simulink model "isi_correlator_tvg"  (Simulink 7.3)
settings: Solver=ode45  StartTime=0.0  StopTime=10.0  RelTol=1e-3
undefined symbols (not defined in the file or in its folder): pfb_coeff_gen_calc, ROACH
libraries: xbsIndex_r4 x5206, casper_library x454, isi_correlator_lib x28, dspsigops x16, xps_library x14
(part 2 of 51)

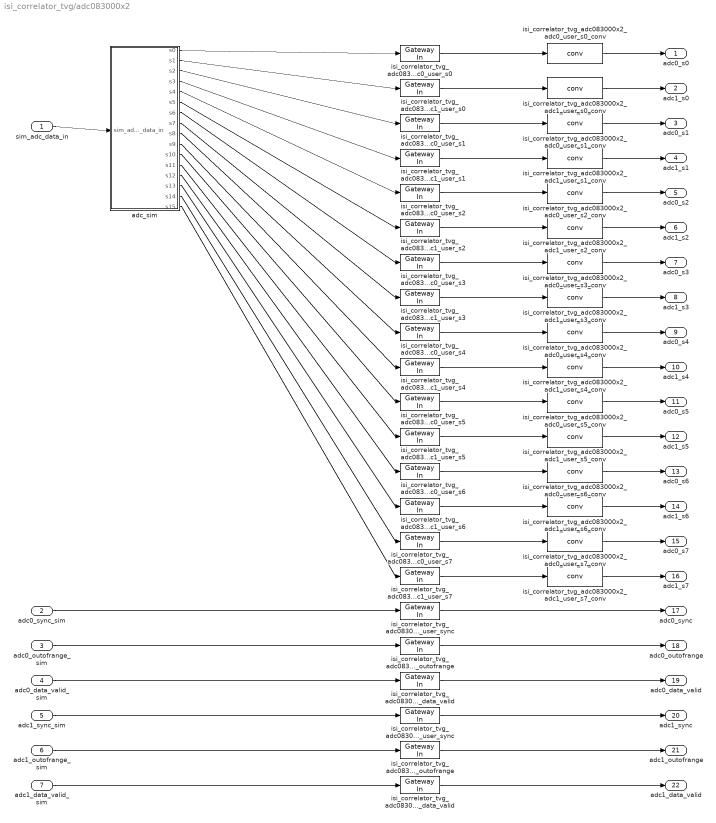
== system isi_correlator_tvg/adc083000x2 ==
  sim_adc_data_in : Inport  OutDataType=sfix(16)  OutScaling=2^0
  adc0_sync_sim : Inport  Port=2  OutDataType=sfix(16)  OutScaling=2^0
  adc0_outofrange_sim : Inport  Port=3  OutDataType=sfix(16)  OutScaling=2^0
  adc0_data_valid_sim : Inport  Port=4  OutDataType=sfix(16)  OutScaling=2^0
  adc1_sync_sim : Inport  Port=5  OutDataType=sfix(16)  OutScaling=2^0
  adc1_outofrange_sim : Inport  Port=6  OutDataType=sfix(16)  OutScaling=2^0
  adc1_data_valid_sim : Inport  Port=7  OutDataType=sfix(16)  OutScaling=2^0
  adc_sim : Subsystem  AncestorBlock=casper_library/Misc/adc_sim  mask(adc_sim){nStreams=16}
      mask prompts: nStreams "Number of Output Streams"
      mask help:
      | This block simulates the behavior of a demuxed ADC, which takes
      | as input a waveform and returns digital samples in parallel at
      | a slower rate.
      |
      | This block is used to simulate the behavior
      mask init:
      | adc_sim_init(gcb, ...
      |     'nStreams', nStreams );
  isi_correlator_tvg_adc083000x2_adc0_user_data_valid : xbsIndex_r4/Gateway In  arith_type=Unsigned  n_bits=1  bin_pt=0  quantization="Round  (unbiased: +/- Inf)"  overflow=Saturate  period=1  dbl_ovrd=off  timing_constraint=None  locs_specified=off  LOCs={}  block_type=gatewayin
      settings: infoedit="Gateway in block.  Converts inputs of type Simulink integer, double and fixed point to  Xilinx fixed point type.<br><br>Hardware notes:  In hardware these blocks become top level input ports."  block_version=11.3
  isi_correlator_tvg_adc083000x2_adc0_user_outofrange : xbsIndex_r4/Gateway In  arith_type=Unsigned  n_bits=4  bin_pt=0  quantization="Round  (unbiased: +/- Inf)"  overflow=Saturate  period=1  dbl_ovrd=off  timing_constraint=None  locs_specified=off  LOCs={}  block_type=gatewayin
      settings: infoedit="Gateway in block.  Converts inputs of type Simulink integer, double and fixed point to  Xilinx fixed point type.<br><br>Hardware notes:  In hardware these blocks become top level input ports."  block_version=11.3
  isi_correlator_tvg_adc083000x2_adc0_user_s{0..7} (8 alike) : xbsIndex_r4/Gateway In  arith_type=Unsigned  n_bits=8  bin_pt=0  quantization="Round  (unbiased: +/- Inf)"  overflow=Saturate  period=1  dbl_ovrd=off  timing_constraint=None  locs_specified=off  LOCs={}  block_type=gatewayin
      settings: infoedit="Gateway in block.  Converts inputs of type Simulink integer, double and fixed point to  Xilinx fixed point type.<br><br>Hardware notes:  In hardware these blocks become top level input ports."  block_version=11.3
  isi_correlator_tvg_adc083000x2_adc0_user_s0_conv : casper_library/Misc/conv  SystemSampleTime=-1
  isi_correlator_tvg_adc083000x2_adc0_user_s1_conv : casper_library/Misc/conv  SystemSampleTime=-1
  isi_correlator_tvg_adc083000x2_adc0_user_s2_conv : casper_library/Misc/conv  SystemSampleTime=-1
  isi_correlator_tvg_adc083000x2_adc0_user_s3_conv : casper_library/Misc/conv  SystemSampleTime=-1
  isi_correlator_tvg_adc083000x2_adc0_user_s4_conv : casper_library/Misc/conv  SystemSampleTime=-1
  isi_correlator_tvg_adc083000x2_adc0_user_s5_conv : casper_library/Misc/conv  SystemSampleTime=-1
  isi_correlator_tvg_adc083000x2_adc0_user_s6_conv : casper_library/Misc/conv  SystemSampleTime=-1
  isi_correlator_tvg_adc083000x2_adc0_user_s7_conv : casper_library/Misc/conv  SystemSampleTime=-1
  isi_correlator_tvg_adc083000x2_adc0_user_sync : xbsIndex_r4/Gateway In  arith_type=Unsigned  n_bits=4  bin_pt=0  quantization="Round  (unbiased: +/- Inf)"  overflow=Saturate  period=1  dbl_ovrd=off  timing_constraint=None  locs_specified=off  LOCs={}  block_type=gatewayin
      settings: infoedit="Gateway in block.  Converts inputs of type Simulink integer, double and fixed point to  Xilinx fixed point type.<br><br>Hardware notes:  In hardware these blocks become top level input ports."  block_version=11.3
  isi_correlator_tvg_adc083000x2_adc1_user_data_valid : xbsIndex_r4/Gateway In  arith_type=Unsigned  n_bits=1  bin_pt=0  quantization="Round  (unbiased: +/- Inf)"  overflow=Saturate  period=1  dbl_ovrd=off  timing_constraint=None  locs_specified=off  LOCs={}  block_type=gatewayin
      settings: infoedit="Gateway in block.  Converts inputs of type Simulink integer, double and fixed point to  Xilinx fixed point type.<br><br>Hardware notes:  In hardware these blocks become top level input ports."  block_version=11.3
  isi_correlator_tvg_adc083000x2_adc1_user_outofrange : xbsIndex_r4/Gateway In  arith_type=Unsigned  n_bits=4  bin_pt=0  quantization="Round  (unbiased: +/- Inf)"  overflow=Saturate  period=1  dbl_ovrd=off  timing_constraint=None  locs_specified=off  LOCs={}  block_type=gatewayin
      settings: infoedit="Gateway in block.  Converts inputs of type Simulink integer, double and fixed point to  Xilinx fixed point type.<br><br>Hardware notes:  In hardware these blocks become top level input ports."  block_version=11.3
  isi_correlator_tvg_adc083000x2_adc1_user_s{0..7} (8 alike) : xbsIndex_r4/Gateway In  arith_type=Unsigned  n_bits=8  bin_pt=0  quantization="Round  (unbiased: +/- Inf)"  overflow=Saturate  period=1  dbl_ovrd=off  timing_constraint=None  locs_specified=off  LOCs={}  block_type=gatewayin
      settings: infoedit="Gateway in block.  Converts inputs of type Simulink integer, double and fixed point to  Xilinx fixed point type.<br><br>Hardware notes:  In hardware these blocks become top level input ports."  block_version=11.3
  isi_correlator_tvg_adc083000x2_adc1_user_s0_conv : casper_library/Misc/conv  SystemSampleTime=-1
  isi_correlator_tvg_adc083000x2_adc1_user_s1_conv : casper_library/Misc/conv  SystemSampleTime=-1
  isi_correlator_tvg_adc083000x2_adc1_user_s2_conv : casper_library/Misc/conv  SystemSampleTime=-1
  isi_correlator_tvg_adc083000x2_adc1_user_s3_conv : casper_library/Misc/conv  SystemSampleTime=-1
  isi_correlator_tvg_adc083000x2_adc1_user_s4_conv : casper_library/Misc/conv  SystemSampleTime=-1
  isi_correlator_tvg_adc083000x2_adc1_user_s5_conv : casper_library/Misc/conv  SystemSampleTime=-1
  isi_correlator_tvg_adc083000x2_adc1_user_s6_conv : casper_library/Misc/conv  SystemSampleTime=-1
  isi_correlator_tvg_adc083000x2_adc1_user_s7_conv : casper_library/Misc/conv  SystemSampleTime=-1
  isi_correlator_tvg_adc083000x2_adc1_user_sync : xbsIndex_r4/Gateway In  arith_type=Unsigned  n_bits=4  bin_pt=0  quantization="Round  (unbiased: +/- Inf)"  overflow=Saturate  period=1  dbl_ovrd=off  timing_constraint=None  locs_specified=off  LOCs={}  block_type=gatewayin
      settings: infoedit="Gateway in block.  Converts inputs of type Simulink integer, double and fixed point to  Xilinx fixed point type.<br><br>Hardware notes:  In hardware these blocks become top level input ports."  block_version=11.3
  adc0_s0 : Outport  OutDataType=fixdt(1, 16)  OutScaling=2^0
  adc1_s0 : Outport  Port=2  OutDataType=fixdt(1, 16)  OutScaling=2^0
  adc0_s1 : Outport  Port=3  OutDataType=fixdt(1, 16)  OutScaling=2^0
  adc1_s1 : Outport  Port=4  OutDataType=fixdt(1, 16)  OutScaling=2^0
  adc0_s2 : Outport  Port=5  OutDataType=fixdt(1, 16)  OutScaling=2^0
  adc1_s2 : Outport  Port=6  OutDataType=fixdt(1, 16)  OutScaling=2^0
  adc0_s3 : Outport  Port=7  OutDataType=fixdt(1, 16)  OutScaling=2^0
  adc1_s3 : Outport  Port=8  OutDataType=fixdt(1, 16)  OutScaling=2^0
  adc0_s4 : Outport  Port=9  OutDataType=fixdt(1, 16)  OutScaling=2^0
  adc1_s4 : Outport  Port=10  OutDataType=fixdt(1, 16)  OutScaling=2^0
  adc0_s5 : Outport  Port=11  OutDataType=fixdt(1, 16)  OutScaling=2^0
  adc1_s5 : Outport  Port=12  OutDataType=fixdt(1, 16)  OutScaling=2^0
  adc0_s6 : Outport  Port=13  OutDataType=fixdt(1, 16)  OutScaling=2^0
  adc1_s6 : Outport  Port=14  OutDataType=fixdt(1, 16)  OutScaling=2^0
  adc0_s7 : Outport  Port=15  OutDataType=fixdt(1, 16)  OutScaling=2^0
  adc1_s7 : Outport  Port=16  OutDataType=fixdt(1, 16)  OutScaling=2^0
  adc0_sync : Outport  Port=17  OutDataType=sfix(16)  OutScaling=2^0
  adc0_outofrange : Outport  Port=18  OutDataType=sfix(16)  OutScaling=2^0
  adc0_data_valid : Outport  Port=19  OutDataType=sfix(16)  OutScaling=2^0
  adc1_sync : Outport  Port=20  OutDataType=sfix(16)  OutScaling=2^0
  adc1_outofrange : Outport  Port=21  OutDataType=sfix(16)  OutScaling=2^0
  adc1_data_valid : Outport  Port=22  OutDataType=sfix(16)  OutScaling=2^0
  wires:
  sim_adc_data_in/1 -> adc_sim/1(sim_adc_data_in)
  adc_sim/1(s0) -> isi_correlator_tvg_adc083000x2_adc0_user_s0/1
  isi_correlator_tvg_adc083000x2_adc0_user_s0/1 -> isi_correlator_tvg_adc083000x2_adc0_user_s0_conv/1
  isi_correlator_tvg_adc083000x2_adc0_user_s0_conv/1 -> adc0_s0/1
  adc_sim/2(s1) -> isi_correlator_tvg_adc083000x2_adc1_user_s0/1
  isi_correlator_tvg_adc083000x2_adc1_user_s0/1 -> isi_correlator_tvg_adc083000x2_adc1_user_s0_conv/1
  isi_correlator_tvg_adc083000x2_adc1_user_s0_conv/1 -> adc1_s0/1
  adc_sim/3(s2) -> isi_correlator_tvg_adc083000x2_adc0_user_s1/1
  isi_correlator_tvg_adc083000x2_adc0_user_s1/1 -> isi_correlator_tvg_adc083000x2_adc0_user_s1_conv/1
  isi_correlator_tvg_adc083000x2_adc0_user_s1_conv/1 -> adc0_s1/1
  adc_sim/4(s3) -> isi_correlator_tvg_adc083000x2_adc1_user_s1/1
  isi_correlator_tvg_adc083000x2_adc1_user_s1/1 -> isi_correlator_tvg_adc083000x2_adc1_user_s1_conv/1
  isi_correlator_tvg_adc083000x2_adc1_user_s1_conv/1 -> adc1_s1/1
  adc_sim/5(s4) -> isi_correlator_tvg_adc083000x2_adc0_user_s2/1
  isi_correlator_tvg_adc083000x2_adc0_user_s2/1 -> isi_correlator_tvg_adc083000x2_adc0_user_s2_conv/1
  isi_correlator_tvg_adc083000x2_adc0_user_s2_conv/1 -> adc0_s2/1
  adc_sim/6(s5) -> isi_correlator_tvg_adc083000x2_adc1_user_s2/1
  isi_correlator_tvg_adc083000x2_adc1_user_s2/1 -> isi_correlator_tvg_adc083000x2_adc1_user_s2_conv/1
  isi_correlator_tvg_adc083000x2_adc1_user_s2_conv/1 -> adc1_s2/1
  adc_sim/7(s6) -> isi_correlator_tvg_adc083000x2_adc0_user_s3/1
  isi_correlator_tvg_adc083000x2_adc0_user_s3/1 -> isi_correlator_tvg_adc083000x2_adc0_user_s3_conv/1
  isi_correlator_tvg_adc083000x2_adc0_user_s3_conv/1 -> adc0_s3/1
  adc_sim/8(s7) -> isi_correlator_tvg_adc083000x2_adc1_user_s3/1
  isi_correlator_tvg_adc083000x2_adc1_user_s3/1 -> isi_correlator_tvg_adc083000x2_adc1_user_s3_conv/1
  isi_correlator_tvg_adc083000x2_adc1_user_s3_conv/1 -> adc1_s3/1
  adc_sim/9(s8) -> isi_correlator_tvg_adc083000x2_adc0_user_s4/1
  isi_correlator_tvg_adc083000x2_adc0_user_s4/1 -> isi_correlator_tvg_adc083000x2_adc0_user_s4_conv/1
  isi_correlator_tvg_adc083000x2_adc0_user_s4_conv/1 -> adc0_s4/1
  adc_sim/10(s9) -> isi_correlator_tvg_adc083000x2_adc1_user_s4/1
  isi_correlator_tvg_adc083000x2_adc1_user_s4/1 -> isi_correlator_tvg_adc083000x2_adc1_user_s4_conv/1
  isi_correlator_tvg_adc083000x2_adc1_user_s4_conv/1 -> adc1_s4/1
  adc_sim/11(s10) -> isi_correlator_tvg_adc083000x2_adc0_user_s5/1
  isi_correlator_tvg_adc083000x2_adc0_user_s5/1 -> isi_correlator_tvg_adc083000x2_adc0_user_s5_conv/1
  isi_correlator_tvg_adc083000x2_adc0_user_s5_conv/1 -> adc0_s5/1
  adc_sim/12(s11) -> isi_correlator_tvg_adc083000x2_adc1_user_s5/1
  isi_correlator_tvg_adc083000x2_adc1_user_s5/1 -> isi_correlator_tvg_adc083000x2_adc1_user_s5_conv/1
  isi_correlator_tvg_adc083000x2_adc1_user_s5_conv/1 -> adc1_s5/1
  adc_sim/13(s12) -> isi_correlator_tvg_adc083000x2_adc0_user_s6/1
  isi_correlator_tvg_adc083000x2_adc0_user_s6/1 -> isi_correlator_tvg_adc083000x2_adc0_user_s6_conv/1
  isi_correlator_tvg_adc083000x2_adc0_user_s6_conv/1 -> adc0_s6/1
  adc_sim/14(s13) -> isi_correlator_tvg_adc083000x2_adc1_user_s6/1
  isi_correlator_tvg_adc083000x2_adc1_user_s6/1 -> isi_correlator_tvg_adc083000x2_adc1_user_s6_conv/1
  isi_correlator_tvg_adc083000x2_adc1_user_s6_conv/1 -> adc1_s6/1
  adc_sim/15(s14) -> isi_correlator_tvg_adc083000x2_adc0_user_s7/1
  isi_correlator_tvg_adc083000x2_adc0_user_s7/1 -> isi_correlator_tvg_adc083000x2_adc0_user_s7_conv/1
  isi_correlator_tvg_adc083000x2_adc0_user_s7_conv/1 -> adc0_s7/1
  adc_sim/16(s15) -> isi_correlator_tvg_adc083000x2_adc1_user_s7/1
  isi_correlator_tvg_adc083000x2_adc1_user_s7/1 -> isi_correlator_tvg_adc083000x2_adc1_user_s7_conv/1
  isi_correlator_tvg_adc083000x2_adc1_user_s7_conv/1 -> adc1_s7/1
  adc0_sync_sim/1 -> isi_correlator_tvg_adc083000x2_adc0_user_sync/1
  isi_correlator_tvg_adc083000x2_adc0_user_sync/1 -> adc0_sync/1
  adc0_outofrange_sim/1 -> isi_correlator_tvg_adc083000x2_adc0_user_outofrange/1
  isi_correlator_tvg_adc083000x2_adc0_user_outofrange/1 -> adc0_outofrange/1
  adc0_data_valid_sim/1 -> isi_correlator_tvg_adc083000x2_adc0_user_data_valid/1
  isi_correlator_tvg_adc083000x2_adc0_user_data_valid/1 -> adc0_data_valid/1
  adc1_sync_sim/1 -> isi_correlator_tvg_adc083000x2_adc1_user_sync/1
  isi_correlator_tvg_adc083000x2_adc1_user_sync/1 -> adc1_sync/1
  adc1_outofrange_sim/1 -> isi_correlator_tvg_adc083000x2_adc1_user_outofrange/1
  isi_correlator_tvg_adc083000x2_adc1_user_outofrange/1 -> adc1_outofrange/1
  adc1_data_valid_sim/1 -> isi_correlator_tvg_adc083000x2_adc1_user_data_valid/1
  isi_correlator_tvg_adc083000x2_adc1_user_data_valid/1 -> adc1_data_valid/1

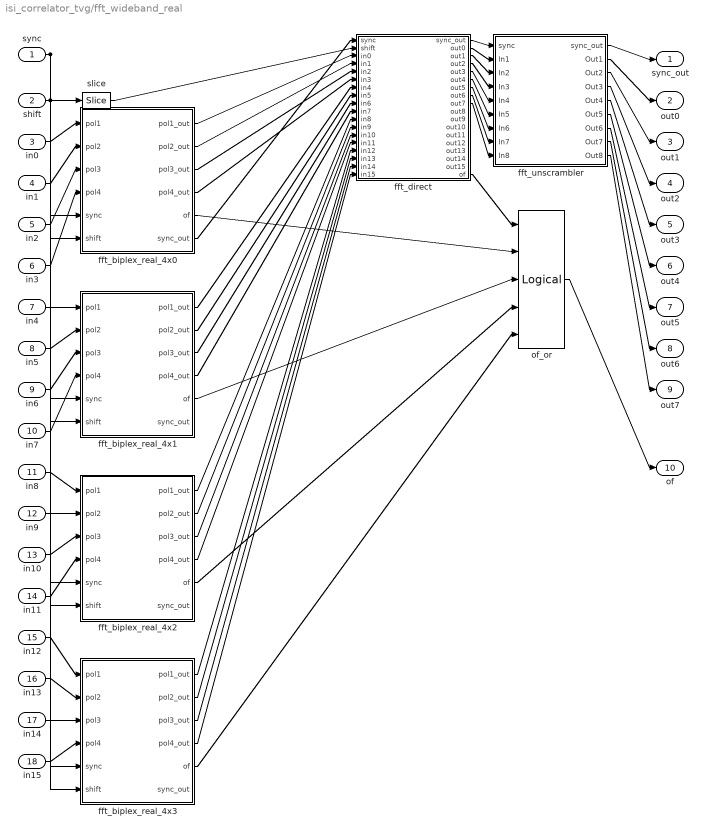
== system isi_correlator_tvg/fft_wideband_real ==
  sync : Inport  OutDataType=sfix(16)  OutScaling=2^0
  shift : Inport  Port=2  OutDataType=sfix(16)  OutScaling=2^0
  in0 : Inport  Port=3  OutDataType=sfix(16)  OutScaling=2^0
  in1 : Inport  Port=4  OutDataType=sfix(16)  OutScaling=2^0
  in2 : Inport  Port=5  OutDataType=sfix(16)  OutScaling=2^0
  in3 : Inport  Port=6  OutDataType=sfix(16)  OutScaling=2^0
  in4 : Inport  Port=7
  in5 : Inport  Port=8
  in6 : Inport  Port=9
  in7 : Inport  Port=10
  in8 : Inport  Port=11
  in9 : Inport  Port=12
  in10 : Inport  Port=13
  in11 : Inport  Port=14
  in12 : Inport  Port=15
  in13 : Inport  Port=16
  in14 : Inport  Port=17
  in15 : Inport  Port=18
  fft_biplex_real_4x0 : Subsystem  AncestorBlock=casper_library/FFTs/fft_biplex_real_4x  mask(fft_biplex_real_4x){FFTSize=3, input_bit_width=18, coeff_bit_width=18, quantization=Truncate, overflow=Wrap, add_latency=1, mult_latency=3, bram_latency=3, arch=Virtex5, opt_target=Multipliers, coeffs_bit_limit=8, delays_bit_limit=9, specify_mult=on, mult_spec=[1 1 1], dsp48_adders=on}
      mask prompts: FFTSize "Size of FFT: (2^? pnts)"; input_bit_width "Input/Output Bit Width"; coeff_bit_width "Coefficient Bit Width"; quantization "Quantization Behavior"; overflow "Overflow Behavior"; add_latency "Add Latency"; mult_latency "Mult Latency"; bram_latency "BRAM Latency"; arch "Architecture"; opt_target "Optimisation Target"; coeffs_bit_limit "Number bits above which to store stage's coefficients in BRAM (2^? bits)"; delays_bit_limit "Number bits above which to implement stage's delays in BRAM (2^? bits)"; specify_mult "Specify multiplier use (behavioral HDL if not specified)"; mult_spec "Multiplier specification (0=core, 1=embedded, 2=behavioural) (left=1st stage)"; dsp48_adders "Use DSP48s for adders"
      mask init:
      | fft_biplex_real_4x_init(gcb, ...
      |     'FFTSize', FFTSize, ...
      |     'input_bit_width', input_bit_width, ...
      |     'coeff_bit_width', coeff_bit_width, ...
      |     'quantization', quantization, ...
      |     'overflow', overflow, ...
      |     'add_latency', add_latency, ...
      |     'mult_latency', mult_latency, ...
      |     'bram_latency', bram_latency, ...
      |     'arch', arch, ...
      |     'opt_target', opt_target, ...
      |     'coeffs_bit_limit', coeffs_bit_limit, ...
      |     'delays_bit_limit', delays_bit_limit, ...    
      |     'specify_mult', specify_mult, ...
      |     'mult_spec', mult_spec, ...
      |     'dsp48_adders', dsp48_adders);
      settings: AttributesFormatString=FFTSize=3
      mask Help: eval('xlWeb([getenv(''MLIB_ROOT''), ''\doc\htmlblocks\sp_fft_biplex_real_4x\sp_fft_biplex_real_4x.html''])')
      mask StyleString: edit,edit,edit,popup(Truncate|Round  (unbiased: +/- Inf)|Round  (unbiased: Even Values)),popup(Wrap|Saturate|Error),edit,edit,edit,popup(Virtex2Pro|Virtex5),popup(Logic|Multipliers),edit,edit,checkbox,edit,checkbox
      mask CallbackString:
      | % fft_size = eval(get_param(gcb, 'FFTSize'));
      | % old_vec = eval(get_param(gcb, 'mult_spec'));
      | 
      | % vec = 2.*ones(1,fft_size);
      | % old_size = length(old_vec);
      | 
      | % if(old_size <= fft_size),
      | %     size = old_size;
      | % else,
      | %     size = fft_size;
      | % end
      | 
      | % for n = 1:size, vec(n) = old_vec(n); end
      | % set_param(gcb, 'mult_spec', mat2str(vec));||||||||||||specify_mult = get_param(gcb,'specify_mult');
      | visibs = get_param(gcb, 'MaskVisibilities');
      | enables = get_param(gcb, 'MaskEnables');
      | enables{14} = 'on';
      | if( strcmp(specify_mult,'off')), visibs{14} = 'off';
      | else, visibs{14} = 'on';
      | end
      | set_param(gcb, 'MaskVisibilities', visibs);
      | set_param(gcb, 'MaskEnables', enables);
      | |% vec = eval( get_param(gcb, 'mult_spec'));
      | % vec_size = length(vec);
      | % fft_size = eval( get_param(gcb, 'FFTSize'));
      | % new_vec = 2.*ones(1, fft_size);
      | 
      | % if(vec_size <= fft_size), size = vec_size;
      | % else size = fft_size;
      | % end
      | 
      | % for n=1:size
      | %    new_vec(n) = vec(n);
      | % end
      | % set_param(gcb, 'mult_spec', mat2str(new_vec));|
  fft_biplex_real_4x1 : Subsystem  AncestorBlock=casper_library/FFTs/fft_biplex_real_4x  mask(fft_biplex_real_4x){FFTSize=3, input_bit_width=18, coeff_bit_width=18, quantization=Truncate, overflow=Wrap, add_latency=1, mult_latency=3, bram_latency=3, arch=Virtex5, opt_target=Multipliers, coeffs_bit_limit=8, delays_bit_limit=9, specify_mult=on, mult_spec=[1 1 1], dsp48_adders=on}
      mask prompts: FFTSize "Size of FFT: (2^? pnts)"; input_bit_width "Input/Output Bit Width"; coeff_bit_width "Coefficient Bit Width"; quantization "Quantization Behavior"; overflow "Overflow Behavior"; add_latency "Add Latency"; mult_latency "Mult Latency"; bram_latency "BRAM Latency"; arch "Architecture"; opt_target "Optimisation Target"; coeffs_bit_limit "Number bits above which to store stage's coefficients in BRAM (2^? bits)"; delays_bit_limit "Number bits above which to implement stage's delays in BRAM (2^? bits)"; specify_mult "Specify multiplier use (behavioral HDL if not specified)"; mult_spec "Multiplier specification (0=core, 1=embedded, 2=behavioural) (left=1st stage)"; dsp48_adders "Use DSP48s for adders"
      mask init: same as isi_correlator_tvg/fft_wideband_real/fft_biplex_real_4x0
      settings: AttributesFormatString=FFTSize=3
      mask Help: eval('xlWeb([getenv(''MLIB_ROOT''), ''\doc\htmlblocks\sp_fft_biplex_real_4x\sp_fft_biplex_real_4x.html''])')
      mask StyleString: edit,edit,edit,popup(Truncate|Round  (unbiased: +/- Inf)|Round  (unbiased: Even Values)),popup(Wrap|Saturate|Error),edit,edit,edit,popup(Virtex2Pro|Virtex5),popup(Logic|Multipliers),edit,edit,checkbox,edit,checkbox
      mask CallbackString:
      | % fft_size = eval(get_param(gcb, 'FFTSize'));
      | % old_vec = eval(get_param(gcb, 'mult_spec'));
      | 
      | % vec = 2.*ones(1,fft_size);
      | % old_size = length(old_vec);
      | 
      | % if(old_size <= fft_size),
      | %     size = old_size;
      | % else,
      | %     size = fft_size;
      | % end
      | 
      | % for n = 1:size, vec(n) = old_vec(n); end
      | % set_param(gcb, 'mult_spec', mat2str(vec));||||||||||||specify_mult = get_param(gcb,'specify_mult');
      | visibs = get_param(gcb, 'MaskVisibilities');
      | enables = get_param(gcb, 'MaskEnables');
      | enables{14} = 'on';
      | if( strcmp(specify_mult,'off')), visibs{14} = 'off';
      | else, visibs{14} = 'on';
      | end
      | set_param(gcb, 'MaskVisibilities', visibs);
      | set_param(gcb, 'MaskEnables', enables);
      | |% vec = eval( get_param(gcb, 'mult_spec'));
      | % vec_size = length(vec);
      | % fft_size = eval( get_param(gcb, 'FFTSize'));
      | % new_vec = 2.*ones(1, fft_size);
      | 
      | % if(vec_size <= fft_size), size = vec_size;
      | % else size = fft_size;
      | % end
      | 
      | % for n=1:size
      | %    new_vec(n) = vec(n);
      | % end
      | % set_param(gcb, 'mult_spec', mat2str(new_vec));|
  fft_biplex_real_4x2 : Subsystem  AncestorBlock=casper_library/FFTs/fft_biplex_real_4x  mask(fft_biplex_real_4x){FFTSize=3, input_bit_width=18, coeff_bit_width=18, quantization=Truncate, overflow=Wrap, add_latency=1, mult_latency=3, bram_latency=3, arch=Virtex5, opt_target=Multipliers, coeffs_bit_limit=8, delays_bit_limit=9, specify_mult=on, mult_spec=[1 1 1], dsp48_adders=on}
      mask prompts: FFTSize "Size of FFT: (2^? pnts)"; input_bit_width "Input/Output Bit Width"; coeff_bit_width "Coefficient Bit Width"; quantization "Quantization Behavior"; overflow "Overflow Behavior"; add_latency "Add Latency"; mult_latency "Mult Latency"; bram_latency "BRAM Latency"; arch "Architecture"; opt_target "Optimisation Target"; coeffs_bit_limit "Number bits above which to store stage's coefficients in BRAM (2^? bits)"; delays_bit_limit "Number bits above which to implement stage's delays in BRAM (2^? bits)"; specify_mult "Specify multiplier use (behavioral HDL if not specified)"; mult_spec "Multiplier specification (0=core, 1=embedded, 2=behavioural) (left=1st stage)"; dsp48_adders "Use DSP48s for adders"
      mask init: same as isi_correlator_tvg/fft_wideband_real/fft_biplex_real_4x0
      settings: AttributesFormatString=FFTSize=3
      mask Help: eval('xlWeb([getenv(''MLIB_ROOT''), ''\doc\htmlblocks\sp_fft_biplex_real_4x\sp_fft_biplex_real_4x.html''])')
      mask StyleString: edit,edit,edit,popup(Truncate|Round  (unbiased: +/- Inf)|Round  (unbiased: Even Values)),popup(Wrap|Saturate|Error),edit,edit,edit,popup(Virtex2Pro|Virtex5),popup(Logic|Multipliers),edit,edit,checkbox,edit,checkbox
      mask CallbackString:
      | % fft_size = eval(get_param(gcb, 'FFTSize'));
      | % old_vec = eval(get_param(gcb, 'mult_spec'));
      | 
      | % vec = 2.*ones(1,fft_size);
      | % old_size = length(old_vec);
      | 
      | % if(old_size <= fft_size),
      | %     size = old_size;
      | % else,
      | %     size = fft_size;
      | % end
      | 
      | % for n = 1:size, vec(n) = old_vec(n); end
      | % set_param(gcb, 'mult_spec', mat2str(vec));||||||||||||specify_mult = get_param(gcb,'specify_mult');
      | visibs = get_param(gcb, 'MaskVisibilities');
      | enables = get_param(gcb, 'MaskEnables');
      | enables{14} = 'on';
      | if( strcmp(specify_mult,'off')), visibs{14} = 'off';
      | else, visibs{14} = 'on';
      | end
      | set_param(gcb, 'MaskVisibilities', visibs);
      | set_param(gcb, 'MaskEnables', enables);
      | |% vec = eval( get_param(gcb, 'mult_spec'));
      | % vec_size = length(vec);
      | % fft_size = eval( get_param(gcb, 'FFTSize'));
      | % new_vec = 2.*ones(1, fft_size);
      | 
      | % if(vec_size <= fft_size), size = vec_size;
      | % else size = fft_size;
      | % end
      | 
      | % for n=1:size
      | %    new_vec(n) = vec(n);
      | % end
      | % set_param(gcb, 'mult_spec', mat2str(new_vec));|
  fft_biplex_real_4x3 : Subsystem  AncestorBlock=casper_library/FFTs/fft_biplex_real_4x  mask(fft_biplex_real_4x){FFTSize=3, input_bit_width=18, coeff_bit_width=18, quantization=Truncate, overflow=Wrap, add_latency=1, mult_latency=3, bram_latency=3, arch=Virtex5, opt_target=Multipliers, coeffs_bit_limit=8, delays_bit_limit=9, specify_mult=on, mult_spec=[1 1 1], dsp48_adders=on}
      mask prompts: FFTSize "Size of FFT: (2^? pnts)"; input_bit_width "Input/Output Bit Width"; coeff_bit_width "Coefficient Bit Width"; quantization "Quantization Behavior"; overflow "Overflow Behavior"; add_latency "Add Latency"; mult_latency "Mult Latency"; bram_latency "BRAM Latency"; arch "Architecture"; opt_target "Optimisation Target"; coeffs_bit_limit "Number bits above which to store stage's coefficients in BRAM (2^? bits)"; delays_bit_limit "Number bits above which to implement stage's delays in BRAM (2^? bits)"; specify_mult "Specify multiplier use (behavioral HDL if not specified)"; mult_spec "Multiplier specification (0=core, 1=embedded, 2=behavioural) (left=1st stage)"; dsp48_adders "Use DSP48s for adders"
      mask init: same as isi_correlator_tvg/fft_wideband_real/fft_biplex_real_4x0
      settings: AttributesFormatString=FFTSize=3
      mask Help: eval('xlWeb([getenv(''MLIB_ROOT''), ''\doc\htmlblocks\sp_fft_biplex_real_4x\sp_fft_biplex_real_4x.html''])')
      mask StyleString: edit,edit,edit,popup(Truncate|Round  (unbiased: +/- Inf)|Round  (unbiased: Even Values)),popup(Wrap|Saturate|Error),edit,edit,edit,popup(Virtex2Pro|Virtex5),popup(Logic|Multipliers),edit,edit,checkbox,edit,checkbox
      mask CallbackString:
      | % fft_size = eval(get_param(gcb, 'FFTSize'));
      | % old_vec = eval(get_param(gcb, 'mult_spec'));
      | 
      | % vec = 2.*ones(1,fft_size);
      | % old_size = length(old_vec);
      | 
      | % if(old_size <= fft_size),
      | %     size = old_size;
      | % else,
      | %     size = fft_size;
      | % end
      | 
      | % for n = 1:size, vec(n) = old_vec(n); end
      | % set_param(gcb, 'mult_spec', mat2str(vec));||||||||||||specify_mult = get_param(gcb,'specify_mult');
      | visibs = get_param(gcb, 'MaskVisibilities');
      | enables = get_param(gcb, 'MaskEnables');
      | enables{14} = 'on';
      | if( strcmp(specify_mult,'off')), visibs{14} = 'off';
      | else, visibs{14} = 'on';
      | end
      | set_param(gcb, 'MaskVisibilities', visibs);
      | set_param(gcb, 'MaskEnables', enables);
      | |% vec = eval( get_param(gcb, 'mult_spec'));
      | % vec_size = length(vec);
      | % fft_size = eval( get_param(gcb, 'FFTSize'));
      | % new_vec = 2.*ones(1, fft_size);
      | 
      | % if(vec_size <= fft_size), size = vec_size;
      | % else size = fft_size;
      | % end
      | 
      | % for n=1:size
      | %    new_vec(n) = vec(n);
      | % end
      | % set_param(gcb, 'mult_spec', mat2str(new_vec));|
  fft_direct : Subsystem  AncestorBlock=casper_library/FFTs/fft_direct  mask(fft_direct){FFTSize=4, input_bit_width=18, coeff_bit_width=18, add_latency=1, mult_latency=3, bram_latency=3, quantization=Truncate, overflow=Wrap, map_tail=on, LargerFFTSize=7, StartStage=4, arch=Virtex5, opt_target=Multipliers, coeffs_bit_limit=8, specify_mult=on, mult_spec=[1 1 1 1], dsp48_adders=on}
      mask prompts: FFTSize "Size of FFT: (2^?)"; input_bit_width "Input/Output Bit Width"; coeff_bit_width "Coefficient Bit Width"; add_latency "Add Latency"; mult_latency "Mult Latency"; bram_latency "BRAM Latency"; quantization "Quantization Behavior"; overflow "Overflow Behavior"; map_tail "Map Tail of Larger FFT"; LargerFFTSize "Size of Larger FFT (2^?):"; StartStage "Start Stage of Map Tail:"; arch "Architecture"; opt_target "Optimisation Target"; coeffs_bit_limit "Number bits above which to store stage's coefficients in BRAM (2^? bits)"; specify_mult "Specify multiplier use (behavioural HDL if not specified)"; mult_spec "Multiplier specification (0=core, 1=behavioural HDL, 2=embedded) (left=1st stage)"; dsp48_adders "Use DSP48s for adders"
      mask help:
      | Implements an N-point FFT with the classic
      | butterfly structure with N inputs and N outputs
      mask init:
      | fft_direct_init(gcb,...
      |     'FFTSize', FFTSize, ...
      |     'input_bit_width', input_bit_width, ...
      |     'coeff_bit_width', coeff_bit_width, ...
      |     'add_latency', add_latency, ...
      |     'mult_latency', mult_latency, ...
      |     'bram_latency', bram_latency, ...
      |     'quantization', quantization, ...
      |     'overflow', overflow, ...
      |     'map_tail', map_tail, ...
      |     'LargerFFTSize', LargerFFTSize, ...
      |     'StartStage', StartStage, ...
      |     'arch', arch, ...
      |     'opt_target', opt_target, ...
      |     'coeffs_bit_limit', coeffs_bit_limit, ...
      |     'specify_mult', specify_mult, ...
      |     'mult_spec', mult_spec, ...
      |     'dsp48_adders', dsp48_adders);
      settings: AttributesFormatString=FFTSize=4
      mask StyleString: edit,edit,edit,edit,edit,edit,popup(Truncate|Round  (unbiased: +/- Inf)|Round  (unbiased: Even Values)),popup(Wrap|Saturate|Error),checkbox,edit,edit,popup(Virtex2Pro|Virtex5),popup(Logic|Multipliers),edit,checkbox,edit,checkbox
      mask CallbackString:
      | % fft_size = eval(get_param(gcb, 'FFTSize'));
      | % old_vec = eval(get_param(gcb, 'mult_spec'));
      | 
      | % vec = 2.*ones(1,fft_size);
      | % old_size = length(old_vec);
      | 
      | % if(old_size <= fft_size),
      | %     size = old_size;
      | % else,
      | %     size = fft_size;
      | % end
      | 
      | % for n = 1:size, vec(n) = old_vec(n); end
      | % set_param(gcb, 'mult_spec', mat2str(vec));||||||||map_tail = get_param(gcb,'map_tail');
      | visibs = get_param(gcb, 'MaskVisibilities');
      | if( strcmp(map_tail,'off')),
      |     visibs{10} = 'off';
      |     visibs{11} = 'off';
      | else,
      |     visibs{10} = 'on';
      |     visibs{11} = 'on';
      | end
      | set_param(gcb, 'MaskVisibilities', visibs);
      | ||||||specify_mult = get_param(gcb,'specify_mult');
      | visibs = get_param(gcb, 'MaskVisibilities');
      | if( strcmp(specify_mult,'off')), visibs{16} = 'off';
      | else, visibs{16} = 'on';
      | end
      | set_param(gcb, 'MaskVisibilities', visibs);
      | |% vec = eval( get_param(gcb, 'mult_spec'));
      | % vec_size = length(vec);
      | % fft_size = eval( get_param(gcb, 'FFTSize'));
      | % new_vec = 2.*ones(1, fft_size);
      | 
      | % if(vec_size <= fft_size), size = vec_size;
      | % else size = fft_size;
      | % end
      | 
      | % for n=1:size
      | %     new_vec(n) = vec(n);
      | % end
      | % set_param(gcb, 'mult_spec', mat2str(new_vec));|
  fft_unscrambler : Subsystem  AncestorBlock=casper_library/FFTs/fft_unscrambler  mask(fft_unscrambler){FFTSize=6, n_inputs=3, bram_latency=3}
      mask prompts: FFTSize "Size of FFT: (2^?)"; n_inputs "Number of Simultaneous Inputs: (2^?)"; bram_latency "BRAM Latency"
      mask init:
      | fft_unscrambler_init(gcb,...
      |     'FFTSize',FFTSize,... 
      |     'n_inputs', n_inputs,...
      |     'bram_latency', bram_latency);
      settings: AttributesFormatString=FFTSize=6, n_inputs=3
  of_or : xbsIndex_r4/Logical  logical_function=OR  inputs=5  en=off  latency=1  precision=Full  arith_type=Unsigned  n_bits=16  bin_pt=0  align_bp=on  dbl_ovrd=off  block_type=logical
  slice : xbsIndex_r4/Slice  nbits=4  boolean_output=off  mode=Lower Bit Location + Width  bit1=0  base1=MSB of Input  bit0=3  base0=LSB of Input  dbl_ovrd=off  block_type=slice
      settings: infoedit="Extracts a given range of bits from each input sample and presents it at the output.  The output type is ordinarily unsigned with binary point at zero, but can be Boolean when the slice is one bit wide.<P><P>Hardware notes: In hardware this block costs nothing."  block_version=10.1.3
  sync_out : Outport
  out0 : Outport  Port=2
  out1 : Outport  Port=3
  out2 : Outport  Port=4
  out3 : Outport  Port=5
  out4 : Outport  Port=6
  out5 : Outport  Port=7
  out6 : Outport  Port=8
  out7 : Outport  Port=9
  of : Outport  Port=10
  wires:
  in0/1 -> fft_biplex_real_4x0/1(pol1)
  in1/1 -> fft_biplex_real_4x0/2(pol2)
  in2/1 -> fft_biplex_real_4x0/3(pol3)
  in3/1 -> fft_biplex_real_4x0/4(pol4)
  shift/1 -> fft_biplex_real_4x0/6(shift), fft_biplex_real_4x1/6(shift), fft_biplex_real_4x2/6(shift), fft_biplex_real_4x3/6(shift), slice/1
  sync/1 -> fft_biplex_real_4x0/5(sync), fft_biplex_real_4x1/5(sync), fft_biplex_real_4x2/5(sync), fft_biplex_real_4x3/5(sync)
  fft_biplex_real_4x0/5(of) -> of_or/2
  in4/1 -> fft_biplex_real_4x1/1(pol1)
  in5/1 -> fft_biplex_real_4x1/2(pol2)
  in6/1 -> fft_biplex_real_4x1/3(pol3)
  in7/1 -> fft_biplex_real_4x1/4(pol4)
  fft_biplex_real_4x1/5(of) -> of_or/3
  in8/1 -> fft_biplex_real_4x2/1(pol1)
  in9/1 -> fft_biplex_real_4x2/2(pol2)
  in10/1 -> fft_biplex_real_4x2/3(pol3)
  in11/1 -> fft_biplex_real_4x2/4(pol4)
  fft_biplex_real_4x2/5(of) -> of_or/4
  in12/1 -> fft_biplex_real_4x3/1(pol1)
  in13/1 -> fft_biplex_real_4x3/2(pol2)
  in14/1 -> fft_biplex_real_4x3/3(pol3)
  in15/1 -> fft_biplex_real_4x3/4(pol4)
  fft_biplex_real_4x3/5(of) -> of_or/5
  slice/1 -> fft_direct/2(shift)
  fft_biplex_real_4x0/6(sync_out) -> fft_direct/1(sync)
  fft_biplex_real_4x0/1(pol1_out) -> fft_direct/3(in0)
  fft_biplex_real_4x0/2(pol2_out) -> fft_direct/4(in1)
  fft_biplex_real_4x0/3(pol3_out) -> fft_direct/5(in2)
  fft_biplex_real_4x0/4(pol4_out) -> fft_direct/6(in3)
  fft_biplex_real_4x1/1(pol1_out) -> fft_direct/7(in4)
  fft_biplex_real_4x1/2(pol2_out) -> fft_direct/8(in5)
  fft_biplex_real_4x1/3(pol3_out) -> fft_direct/9(in6)
  fft_biplex_real_4x1/4(pol4_out) -> fft_direct/10(in7)
  fft_biplex_real_4x2/1(pol1_out) -> fft_direct/11(in8)
  fft_biplex_real_4x2/2(pol2_out) -> fft_direct/12(in9)
  fft_biplex_real_4x2/3(pol3_out) -> fft_direct/13(in10)
  fft_biplex_real_4x2/4(pol4_out) -> fft_direct/14(in11)
  fft_biplex_real_4x3/1(pol1_out) -> fft_direct/15(in12)
  fft_biplex_real_4x3/2(pol2_out) -> fft_direct/16(in13)
  fft_biplex_real_4x3/3(pol3_out) -> fft_direct/17(in14)
  fft_biplex_real_4x3/4(pol4_out) -> fft_direct/18(in15)
  fft_direct/18(of) -> of_or/1
  of_or/1 -> of/1
  fft_direct/1(sync_out) -> fft_unscrambler/1(sync)
  fft_unscrambler/1(sync_out) -> sync_out/1
  fft_direct/2(out0) -> fft_unscrambler/2(In1)
  fft_unscrambler/2(Out1) -> out0/1
  fft_direct/3(out1) -> fft_unscrambler/3(In2)
  fft_unscrambler/3(Out2) -> out1/1
  fft_direct/4(out2) -> fft_unscrambler/4(In3)
  fft_unscrambler/4(Out3) -> out2/1
  fft_direct/5(out3) -> fft_unscrambler/5(In4)
  fft_unscrambler/5(Out4) -> out3/1
  fft_direct/6(out4) -> fft_unscrambler/6(In5)
  fft_unscrambler/6(Out5) -> out4/1
  fft_direct/7(out5) -> fft_unscrambler/7(In6)
  fft_unscrambler/7(Out6) -> out5/1
  fft_direct/8(out6) -> fft_unscrambler/8(In7)
  fft_unscrambler/8(Out7) -> out6/1
  fft_direct/9(out7) -> fft_unscrambler/9(In8)
  fft_unscrambler/9(Out8) -> out7/1
